simulink model "TosAeb"  (R2021a)
settings: Solver=FixedStepDiscrete  StartTime=0.0  StopTime=inf  FixedStep=0.01  RelTol=1e-3
undefined symbols (not defined in the file or in its folder): ACCModSts, AccSts1, AEB_ObjectMovingOrientation, AEB_ObjectMovingState, CN_BTCActive, CN_CurrentGearR, CN_CurrentGearR_DHT, CN_PTCActive, CN_VDCActive, CN_VSC_EspOff, CN_VSC_FCW_AEB_Error, CN_VSC_FCW_AEB_NoDispay, CN_VSC_FCW_AEB_PerfmDgdtn, CN_VSC_FCW_AEB_TempUnAvlbl, CN_VSC_VehStandstill, CNaccelAvoidMax, CNaccelAvoidMin, CNbrakePedalPress, CNcomfortStrLeftAccelMax, CNcomfortStrLeftAccelMin, CNcomfortStrRightAccelMax, CNcomfortStrRightAccelMin, CNDrvDoorOpen, CNDrvModeEco, CNDrvModeNorm, CNDrvModeOther, CNDrvModeSport, CNDrvSBRreminder, CNNull, CNobjInvalidID, CNPassDoorOpen, CNPassSBRreminder, CNPEBSAccelNegInf, CNPEBSAccelNull, CNPEBSTimeInf, CNPEBSTimeNull, CNSTOPMAX, CNSTOPNULL, CNsubjWidth, CNSwtOFF, CNSwtON, CNTTCMAX, CNTTCNULL, CNVehConfigAEBCycNone, CNVehConfigAEBNone, CNVehConfigAEBPedNone, CNVehConfigFCWNone, CNVehConfigJANone, CollisionSeverity, FALSE, FCWSnvty, JACycObjCalcInfoDefaultParams, JAPedObjCalcInfoDefaultParams, JAVehObjCalcInfoDefaultParams, latCycObjCalcInfoDefaultParams, latPedObjCalcInfoDefaultParams, longObjCalcInfoDefaultParams, ModSts, movingStatus, ObjectType ... (+1112)
libraries: simulink x3031, AEBSFunctionLib x50, Ta_Library x33, AEBSStateMachineLib x23, Mylibrary x12, simulink_extras x7, ObjSelect_lib x3, dspstat3 x2
(part 33 of 102)

== system TosAeb/FcwAebJa_Step_sys/ALG_AEBSystem/ALG_AEBController/AEB_StateMachineCalcApp/VehFCTModStsArb/BrkReadyArb/BrkReadyModStsArb ==
  vehAEBModSts : Inport
  prefillBrkReadyAbor : Inport  Port=2
  ABABrkReadyAbor : Inport  Port=3
  Bus Selector : BusSelector  OutputSignals=vehPrefillModSts
  Bus Selector1 : BusSelector  OutputSignals=vehABAModSts
  Compare To Constant : simulink/Logic and Bit Operations/Compare To Constant  relop=~=  const=ModSts.ActiveState  OutDataTypeStr=boolean
  Compare To Constant1 : simulink/Logic and Bit Operations/Compare To Constant  relop===  const=FALSE  OutDataTypeStr=boolean
  Compare To Constant2 : simulink/Logic and Bit Operations/Compare To Constant  relop=~=  const=ModSts.ActiveState  OutDataTypeStr=boolean
  Compare To Constant3 : simulink/Logic and Bit Operations/Compare To Constant  relop===  const=FALSE  OutDataTypeStr=boolean
  Constant : Constant  Value=ModSts.StandbyState
  Constant1 : Constant  Value=ModSts.StandbyState
  Switch{,1..3} (4 alike) : Switch  Criteria=u2 ~= 0
  prefillModStsArb : Outport
  ABAModStsArb : Outport  Port=2
  wires:
  vehAEBModSts/1 -> Bus Selector1/1, Bus Selector/1
  Bus Selector/1 -> Switch/1, Switch1/1, Compare To Constant/1  "<vehPrefillModSts>"
  Compare To Constant/1 -> Switch/2
  Switch1/1 -> Switch/3
  Compare To Constant1/1 -> Switch1/2
  prefillBrkReadyAbor/1 -> Compare To Constant1/1
  Constant/1 -> Switch1/3
  Switch/1 -> prefillModStsArb/1
  Bus Selector1/1 -> Switch3/1, Switch2/1, Compare To Constant2/1  "<vehABAModSts>"
  Compare To Constant2/1 -> Switch2/2
  ABABrkReadyAbor/1 -> Compare To Constant3/1
  Compare To Constant3/1 -> Switch3/2
  Constant1/1 -> Switch3/3
  Switch3/1 -> Switch2/3
  Switch2/1 -> ABAModStsArb/1

== system TosAeb/FcwAebJa_Step_sys/ALG_AEBSystem/ALG_AEBController/AEB_StateMachineCalcApp/VehFCTModStsArb/BrkReadyArb/BrkReadySuppArb ==
  brkActvCond : Inport
  Compare To Constant : simulink/Logic and Bit Operations/Compare To Constant  relop===  const=TRUE  OutDataTypeStr=boolean
  Constant : Constant  Value=FALSE  OutDataTypeStr=boolean
  prefillBrkReadySuppArb : Outport
  ABABrkReadySuppArb : Outport  Port=2
  wires:
  brkActvCond/1 -> Compare To Constant/1
  Compare To Constant/1 -> prefillBrkReadySuppArb/1
  Constant/1 -> ABABrkReadySuppArb/1

== system TosAeb/FcwAebJa_Step_sys/ALG_AEBSystem/ALG_AEBController/AEB_StateMachineCalcApp/VehFCTModStsArb/WarningArb/BrkReadyAborArb ==
  brkActvCond : Inport
  brkReadyActvCond : Inport  Port=2
  vehAEBModSts : Inport  Port=3
  Bus Selector : BusSelector  OutputSignals=vehPreWarningModSts,vehAcuteWarningModSts
  Bus Selector1 : BusSelector  OutputSignals=vehEBAModSts
  Compare To Constant : simulink/Logic and Bit Operations/Compare To Constant  relop===  const=TRUE  OutDataTypeStr=boolean
  Compare To Constant1 : simulink/Logic and Bit Operations/Compare To Constant  relop===  const=TRUE  OutDataTypeStr=boolean
  Compare To Constant2 : simulink/Logic and Bit Operations/Compare To Constant  relop===  const=ModSts.ActiveState  OutDataTypeStr=boolean
  Compare To Constant3 : simulink/Logic and Bit Operations/Compare To Constant  relop===  const=ModSts.ActiveState  OutDataTypeStr=boolean
  Compare To Constant4 : simulink/Logic and Bit Operations/Compare To Constant  relop===  const=ModSts.ActiveState  OutDataTypeStr=boolean
  Compare To Constant5 : simulink/Logic and Bit Operations/Compare To Constant  relop===  const=TRUE  OutDataTypeStr=boolean
  Logical Operator : Logic  Operator=OR  Inputs=4  AllPortsSameDT=off  OutDataTypeStr=boolean
  latentWarningAborArb : Outport
  preWarningAborArb : Outport  Port=2
  acuteWarningAborArb : Outport  Port=3
  wires:
  brkReadyActvCond/1 -> Compare To Constant1/1
  vehAEBModSts/1 -> Bus Selector/1, Bus Selector1/1
  Bus Selector/1 -> Compare To Constant2/1  "<vehPreWarningModSts>"
  Bus Selector/2 -> Compare To Constant3/1  "<vehAcuteWarningModSts>"
  Compare To Constant1/1 -> Logical Operator/4
  Compare To Constant/1 -> Logical Operator/3
  Compare To Constant3/1 -> Logical Operator/2
  Compare To Constant2/1 -> Logical Operator/1
  Logical Operator/1 -> latentWarningAborArb/1
  Bus Selector1/1 -> Compare To Constant4/1  "<vehEBAModSts>"
  Compare To Constant4/1 -> preWarningAborArb/1
  brkActvCond/1 -> Compare To Constant5/1, Compare To Constant/1
  Compare To Constant5/1 -> acuteWarningAborArb/1

== system TosAeb/FcwAebJa_Step_sys/ALG_AEBSystem/ALG_AEBController/AEB_StateMachineCalcApp/VehFCTModStsArb/WarningArb/WarningActvArbtrtn ==
  latentWarningModStsArb : Inport
  preWarningModStsArb : Inport  Port=2
  acuteWarningModStsArb : Inport  Port=3
  Compare To Constant : simulink/Logic and Bit Operations/Compare To Constant  relop===  const=ModSts.ActiveState  OutDataTypeStr=boolean
  Compare To Constant1 : simulink/Logic and Bit Operations/Compare To Constant  relop===  const=ModSts.ActiveState  OutDataTypeStr=boolean
  Compare To Constant2 : simulink/Logic and Bit Operations/Compare To Constant  relop===  const=ModSts.ActiveState  OutDataTypeStr=boolean
  Logical Operator : Logic  Operator=OR  Inputs=3  AllPortsSameDT=off  OutDataTypeStr=boolean
  WarningActvCond : Outport
  wires:
  Logical Operator/1 -> WarningActvCond/1
  Compare To Constant/1 -> Logical Operator/1
  Compare To Constant1/1 -> Logical Operator/2
  Compare To Constant2/1 -> Logical Operator/3
  latentWarningModStsArb/1 -> Compare To Constant/1
  preWarningModStsArb/1 -> Compare To Constant1/1
  acuteWarningModStsArb/1 -> Compare To Constant2/1

== system TosAeb/FcwAebJa_Step_sys/ALG_AEBSystem/ALG_AEBController/AEB_StateMachineCalcApp/VehFCTModStsArb/WarningArb/WarningModStsArbtrtn ==
  vehAEBModSts : Inport
  latentWarningAborArb : Inport  Port=2
  preWarningAborArb : Inport  Port=3
  acuteWarningAborArb : Inport  Port=4
  Bus Selector : BusSelector  OutputSignals=vehLatentWarningModSts
  Bus Selector1 : BusSelector  OutputSignals=vehPreWarningModSts
  Bus Selector2 : BusSelector  OutputSignals=vehAcuteWarningModSts
  Compare To Constant : simulink/Logic and Bit Operations/Compare To Constant  relop=~=  const=ModSts.ActiveState  OutDataTypeStr=boolean
  Compare To Constant1 : simulink/Logic and Bit Operations/Compare To Constant  relop===  const=FALSE  OutDataTypeStr=boolean
  Compare To Constant2 : simulink/Logic and Bit Operations/Compare To Constant  relop=~=  const=ModSts.ActiveState  OutDataTypeStr=boolean
  Compare To Constant3 : simulink/Logic and Bit Operations/Compare To Constant  relop===  const=FALSE  OutDataTypeStr=boolean
  Compare To Constant4 : simulink/Logic and Bit Operations/Compare To Constant  relop=~=  const=ModSts.ActiveState  OutDataTypeStr=boolean
  Compare To Constant5 : simulink/Logic and Bit Operations/Compare To Constant  relop===  const=FALSE  OutDataTypeStr=boolean
  Constant : Constant  Value=ModSts.StandbyState
  Constant1 : Constant  Value=ModSts.StandbyState
  Constant2 : Constant  Value=ModSts.StandbyState
  Switch{,1..5} (6 alike) : Switch  Criteria=u2 ~= 0
  latentWarningModStsArb : Outport
  preWarningModStsArb : Outport  Port=2
  acuteWarningModStsArb : Outport  Port=3
  wires:
  Switch2/1 -> preWarningModStsArb/1
  Switch3/1 -> Switch2/3
  Constant1/1 -> Switch3/3
  Compare To Constant3/1 -> Switch3/2
  preWarningAborArb/1 -> Compare To Constant3/1
  Compare To Constant2/1 -> Switch2/2
  Bus Selector1/1 -> Compare To Constant2/1, Switch2/1, Switch3/1  "<vehPreWarningModSts>"
  Switch/1 -> latentWarningModStsArb/1
  Constant/1 -> Switch1/3
  latentWarningAborArb/1 -> Compare To Constant1/1
  Compare To Constant1/1 -> Switch1/2
  Switch1/1 -> Switch/3
  Compare To Constant/1 -> Switch/2
  Bus Selector/1 -> Compare To Constant/1, Switch1/1, Switch/1  "<vehLatentWarningModSts>"
  vehAEBModSts/1 -> Bus Selector/1, Bus Selector2/1, Bus Selector1/1
  Bus Selector2/1 -> Switch5/1, Switch4/1, Compare To Constant4/1  "<vehAcuteWarningModSts>"
  Compare To Constant4/1 -> Switch4/2
  acuteWarningAborArb/1 -> Compare To Constant5/1
  Compare To Constant5/1 -> Switch5/2
  Constant2/1 -> Switch5/3
  Switch5/1 -> Switch4/3
  Switch4/1 -> acuteWarningModStsArb/1

== system TosAeb/FcwAebJa_Step_sys/ALG_AEBSystem/ALG_AEBController/AEB_StateMachineCalcApp/VehFCTModStsArb/WarningArb/WarningSuppArb ==
  vehAEBModSts : Inport
  brkReadyActvCond : Inport  Port=2
  brkActvCond : Inport  Port=3
  Bus Selector : BusSelector  OutputSignals=vehPreWarningModSts,vehLatentWarningModSts
  Compare To Constant{,1,4..5} (4 alike) : simulink/Logic and Bit Operations/Compare To Constant  relop===  const=TRUE  OutDataTypeStr=boolean
  Compare To Constant2 : simulink/Logic and Bit Operations/Compare To Constant  relop===  const=ModSts.ActiveState  OutDataTypeStr=boolean
  Compare To Constant3 : simulink/Logic and Bit Operations/Compare To Constant  relop===  const=ModSts.ActiveState  OutDataTypeStr=boolean
  Logical Operator : Logic  Operator=OR  Inputs=4  AllPortsSameDT=off  OutDataTypeStr=boolean
  latentWarningSuppArb : Outport
  prewarningSuppArb : Outport  Port=2
  acutewarningSuppArb : Outport  Port=3
  wires:
  brkReadyActvCond/1 -> Compare To Constant/1
  brkActvCond/1 -> Compare To Constant5/1, Compare To Constant4/1, Compare To Constant1/1
  vehAEBModSts/1 -> Bus Selector/1
  Bus Selector/1 -> Compare To Constant2/1  "<vehPreWarningModSts>"
  Bus Selector/2 -> Compare To Constant3/1  "<vehLatentWarningModSts>"
  Compare To Constant2/1 -> Logical Operator/1
  Compare To Constant3/1 -> Logical Operator/2
  Compare To Constant/1 -> Logical Operator/3
  Compare To Constant1/1 -> Logical Operator/4
  Logical Operator/1 -> latentWarningSuppArb/1
  Compare To Constant4/1 -> prewarningSuppArb/1
  Compare To Constant5/1 -> acutewarningSuppArb/1

== system TosAeb/FcwAebJa_Step_sys/ALG_AEBSystem/ALG_AEBController/AEB_VehicleStateCalcApp/CycSuppAborCondtionCalc/CycAEBfullSuppAborConditonCalc/VehStateCalcCycAEBfullAborCalc ==
  VSCAEB_PedResp : Inport
  FCW_AEB_PedFctSts : Inport  Port=2
  vehAssociatedSysStateSuppCond : Inport  Port=3
  vehUnstableStateFlg : Inport  Port=4
  Compare To Constant : simulink/Logic and Bit Operations/Compare To Constant  relop===  const=CNSwtOFF  OutDataTypeStr=boolean
  Compare To Constant2 : simulink/Logic and Bit Operations/Compare To Constant  relop===  const=TRUE  OutDataTypeStr=boolean
  Compare To Constant3 : simulink/Logic and Bit Operations/Compare To Constant  relop===  const=TRUE  OutDataTypeStr=boolean
  Compare To Constant4 : simulink/Logic and Bit Operations/Compare To Constant  relop===  const=CN_VSC_FCW_AEB_Error  OutDataTypeStr=boolean
  Compare To Constant8 : simulink/Logic and Bit Operations/Compare To Constant  relop===  const=CN_VSC_FCW_AEB_PerfmDgdtn  OutDataTypeStr=boolean
  Compare To Constant9 : simulink/Logic and Bit Operations/Compare To Constant  relop===  const=CN_VSC_FCW_AEB_TempUnAvlbl  OutDataTypeStr=boolean
  Logical Operator : Logic  Operator=OR  Inputs=3  AllPortsSameDT=off  OutDataTypeStr=boolean
  Logical Operator1 : Logic  Operator=OR  Inputs=4  AllPortsSameDT=off  OutDataTypeStr=boolean
  VSCCycAEBfullAborCond : Outport
  wires:
  FCW_AEB_PedFctSts/1 -> Compare To Constant4/1, Compare To Constant8/1, Compare To Constant9/1
  Logical Operator/1 -> Logical Operator1/2
  Compare To Constant9/1 -> Logical Operator/3
  Compare To Constant8/1 -> Logical Operator/2
  Compare To Constant4/1 -> Logical Operator/1
  vehUnstableStateFlg/1 -> Compare To Constant3/1
  Logical Operator1/1 -> VSCCycAEBfullAborCond/1
  vehAssociatedSysStateSuppCond/1 -> Compare To Constant2/1
  Compare To Constant/1 -> Logical Operator1/1
  VSCAEB_PedResp/1 -> Compare To Constant/1
  Compare To Constant2/1 -> Logical Operator1/3
  Compare To Constant3/1 -> Logical Operator1/4

== system TosAeb/FcwAebJa_Step_sys/ALG_AEBSystem/ALG_AEBController/AEB_VehicleStateCalcApp/CycSuppAborCondtionCalc/CycAEBfullSuppAborConditonCalc/VehStateCalcCycAEBfullSuppCalc ==
  VSCAEB_PedResp : Inport
  FCW_AEB_PedFctSts : Inport  Port=2
  vehUnstableStateFlg : Inport  Port=3
  vehAssociatedSysStateSuppCond : Inport  Port=4
  "vehConfigAEBCyc " : Inport  Port=5
  blockTimeBrakeReady : Inport  Port=6
  Compare To Constant : simulink/Logic and Bit Operations/Compare To Constant  relop===  const=CNSwtOFF  OutDataTypeStr=boolean
  Compare To Constant1 : simulink/Logic and Bit Operations/Compare To Constant  relop===  const=CN_VSC_FCW_AEB_Error  OutDataTypeStr=boolean
  Compare To Constant3 : simulink/Logic and Bit Operations/Compare To Constant  relop===  const=CNVehConfigAEBCycNone  OutDataTypeStr=boolean
  Compare To Constant4 : simulink/Logic and Bit Operations/Compare To Constant  relop=>  const=CNNull  OutDataTypeStr=boolean
  Compare To Constant5 : simulink/Logic and Bit Operations/Compare To Constant  relop===  const=TRUE  OutDataTypeStr=boolean
  Compare To Constant6 : simulink/Logic and Bit Operations/Compare To Constant  relop===  const=TRUE  OutDataTypeStr=boolean
  Compare To Constant7 : simulink/Logic and Bit Operations/Compare To Constant  relop===  const=CNSwtOFF  OutDataTypeStr=boolean
  Compare To Constant8 : simulink/Logic and Bit Operations/Compare To Constant  relop===  const=CN_VSC_FCW_AEB_PerfmDgdtn  OutDataTypeStr=boolean
  Compare To Constant9 : simulink/Logic and Bit Operations/Compare To Constant  relop===  const=CN_VSC_FCW_AEB_TempUnAvlbl  OutDataTypeStr=boolean
  Constant : Constant  Value=P_AEB_InterSwtSts4CycAEBfull
  Logical Operator : Logic  Operator=OR  Inputs=3  AllPortsSameDT=off  OutDataTypeStr=boolean
  Logical Operator1 : Logic  Operator=OR  Inputs=7  AllPortsSameDT=off  OutDataTypeStr=boolean
  VSCCycAEBfullSuppCond : Outport
  wires:
  FCW_AEB_PedFctSts/1 -> Compare To Constant1/1, Compare To Constant8/1, Compare To Constant9/1
  Compare To Constant1/1 -> Logical Operator/1
  Compare To Constant9/1 -> Logical Operator/3
  Compare To Constant8/1 -> Logical Operator/2
  Compare To Constant7/1 -> Logical Operator1/7
  Constant/1 -> Compare To Constant7/1
  VSCAEB_PedResp/1 -> Compare To Constant/1
  Compare To Constant/1 -> Logical Operator1/1
  Compare To Constant3/1 -> Logical Operator1/5
  vehAssociatedSysStateSuppCond/1 -> Compare To Constant5/1
  blockTimeBrakeReady/1 -> Compare To Constant4/1
  Logical Operator/1 -> Logical Operator1/2
  vehUnstableStateFlg/1 -> Compare To Constant6/1
  Logical Operator1/1 -> VSCCycAEBfullSuppCond/1
  "vehConfigAEBCyc "/1 -> Compare To Constant3/1
  Compare To Constant4/1 -> Logical Operator1/6
  Compare To Constant5/1 -> Logical Operator1/4
  Compare To Constant6/1 -> Logical Operator1/3

== system TosAeb/FcwAebJa_Step_sys/ALG_AEBSystem/ALG_AEBController/AEB_VehicleStateCalcApp/CycSuppAborCondtionCalc/CycAEBpartSuppAborConditonCalc/VehStateCalcCycAEBpartAborCalc ==
  AEB_PedResp : Inport
  FCW_AEB_PedFctSts : Inport  Port=2
  vehAssociatedSysStateSuppCond : Inport  Port=3
  vehUnstableStateFlg : Inport  Port=4
  Compare To Constant : simulink/Logic and Bit Operations/Compare To Constant  relop===  const=CNSwtOFF  OutDataTypeStr=boolean
  Compare To Constant2 : simulink/Logic and Bit Operations/Compare To Constant  relop===  const=TRUE  OutDataTypeStr=boolean
  Compare To Constant3 : simulink/Logic and Bit Operations/Compare To Constant  relop===  const=TRUE  OutDataTypeStr=boolean
  Compare To Constant6 : simulink/Logic and Bit Operations/Compare To Constant  relop===  const=CN_VSC_FCW_AEB_Error  OutDataTypeStr=boolean
  Compare To Constant8 : simulink/Logic and Bit Operations/Compare To Constant  relop===  const=CN_VSC_FCW_AEB_PerfmDgdtn  OutDataTypeStr=boolean
  Compare To Constant9 : simulink/Logic and Bit Operations/Compare To Constant  relop===  const=CN_VSC_FCW_AEB_TempUnAvlbl  OutDataTypeStr=boolean
  Logical Operator : Logic  Operator=OR  Inputs=3  AllPortsSameDT=off  OutDataTypeStr=boolean
  Logical Operator1 : Logic  Operator=OR  Inputs=4  AllPortsSameDT=off  OutDataTypeStr=boolean
  VSCAEBpartAborCond : Outport
  wires:
  FCW_AEB_PedFctSts/1 -> Compare To Constant6/1, Compare To Constant8/1, Compare To Constant9/1
  Compare To Constant6/1 -> Logical Operator/1
  Compare To Constant9/1 -> Logical Operator/3
  Compare To Constant8/1 -> Logical Operator/2
  AEB_PedResp/1 -> Compare To Constant/1
  Compare To Constant/1 -> Logical Operator1/1
  vehAssociatedSysStateSuppCond/1 -> Compare To Constant2/1
  Logical Operator/1 -> Logical Operator1/2
  Logical Operator1/1 -> VSCAEBpartAborCond/1
  vehUnstableStateFlg/1 -> Compare To Constant3/1
  Compare To Constant2/1 -> Logical Operator1/3
  Compare To Constant3/1 -> Logical Operator1/4

== system TosAeb/FcwAebJa_Step_sys/ALG_AEBSystem/ALG_AEBController/AEB_VehicleStateCalcApp/CycSuppAborCondtionCalc/CycAEBpartSuppAborConditonCalc/VehStateCalcCycAEBpartSuppCalc ==
  AEB_PedResp : Inport
  FCW_AEB_PedFctSts : Inport  Port=2
  vehUnstableStateFlg : Inport  Port=3
  vehAssociatedSysStateSuppCond : Inport  Port=4
  "vehConfigAEBCyc " : Inport  Port=5
  blockTimeBrake : Inport  Port=6
  Compare To Constant : simulink/Logic and Bit Operations/Compare To Constant  relop===  const=CNSwtOFF  OutDataTypeStr=boolean
  Compare To Constant2 : simulink/Logic and Bit Operations/Compare To Constant  relop===  const=TRUE  OutDataTypeStr=boolean
  Compare To Constant3 : simulink/Logic and Bit Operations/Compare To Constant  relop===  const=CNVehConfigAEBCycNone  OutDataTypeStr=boolean
  Compare To Constant4 : simulink/Logic and Bit Operations/Compare To Constant  relop=>  const=CNNull  OutDataTypeStr=boolean
  Compare To Constant5 : simulink/Logic and Bit Operations/Compare To Constant  relop===  const=TRUE  OutDataTypeStr=boolean
  Compare To Constant6 : simulink/Logic and Bit Operations/Compare To Constant  relop===  const=CN_VSC_FCW_AEB_Error  OutDataTypeStr=boolean
  Compare To Constant7 : simulink/Logic and Bit Operations/Compare To Constant  relop===  const=CNSwtOFF  OutDataTypeStr=boolean
  Compare To Constant8 : simulink/Logic and Bit Operations/Compare To Constant  relop===  const=CN_VSC_FCW_AEB_PerfmDgdtn  OutDataTypeStr=boolean
  Compare To Constant9 : simulink/Logic and Bit Operations/Compare To Constant  relop===  const=CN_VSC_FCW_AEB_TempUnAvlbl  OutDataTypeStr=boolean
  Constant : Constant  Value=P_AEB_InterSwtSts4CycAEBpart
  Logical Operator : Logic  Operator=OR  Inputs=3  AllPortsSameDT=off  OutDataTypeStr=boolean
  Logical Operator1 : Logic  Operator=OR  Inputs=7  AllPortsSameDT=off  OutDataTypeStr=boolean
  VSCAEBpartSuppCond : Outport
  wires:
  FCW_AEB_PedFctSts/1 -> Compare To Constant6/1, Compare To Constant8/1, Compare To Constant9/1
  Logical Operator/1 -> Logical Operator1/2
  Compare To Constant6/1 -> Logical Operator/1
  Compare To Constant8/1 -> Logical Operator/2
  Compare To Constant9/1 -> Logical Operator/3
  Compare To Constant7/1 -> Logical Operator1/7
  Constant/1 -> Compare To Constant7/1
  Compare To Constant4/1 -> Logical Operator1/6
  "vehConfigAEBCyc "/1 -> Compare To Constant3/1
  Logical Operator1/1 -> VSCAEBpartSuppCond/1
  vehUnstableStateFlg/1 -> Compare To Constant5/1
  blockTimeBrake/1 -> Compare To Constant4/1
  vehAssociatedSysStateSuppCond/1 -> Compare To Constant2/1
  Compare To Constant3/1 -> Logical Operator1/5
  Compare To Constant/1 -> Logical Operator1/1
  AEB_PedResp/1 -> Compare To Constant/1
  Compare To Constant2/1 -> Logical Operator1/4
  Compare To Constant5/1 -> Logical Operator1/3

== system TosAeb/FcwAebJa_Step_sys/ALG_AEBSystem/ALG_AEBController/AEB_VehicleStateCalcApp/CycSuppAborCondtionCalc/CycPrefillSuppAborConditonCalc/VehStateCalcPrefillAborCalc ==
  AEB_PedResp : Inport
  FCW_AEB_PedFctSts : Inport  Port=2
  vehUnstableStateFlg : Inport  Port=3
  vehAssociatedSysStateSuppCond : Inport  Port=4
  Compare To Constant : simulink/Logic and Bit Operations/Compare To Constant  relop===  const=CNSwtOFF  OutDataTypeStr=boolean
  Compare To Constant2 : simulink/Logic and Bit Operations/Compare To Constant  relop===  const=TRUE  OutDataTypeStr=boolean
  Compare To Constant3 : simulink/Logic and Bit Operations/Compare To Constant  relop===  const=TRUE  OutDataTypeStr=boolean
  Compare To Constant4 : simulink/Logic and Bit Operations/Compare To Constant  relop===  const=CN_VSC_FCW_AEB_Error  OutDataTypeStr=boolean
  Compare To Constant5 : simulink/Logic and Bit Operations/Compare To Constant  relop===  const=CN_VSC_FCW_AEB_PerfmDgdtn  OutDataTypeStr=boolean
  Compare To Constant8 : simulink/Logic and Bit Operations/Compare To Constant  relop===  const=CN_VSC_FCW_AEB_TempUnAvlbl  OutDataTypeStr=boolean
  Logical Operator : Logic  Operator=OR  Inputs=3  AllPortsSameDT=off  OutDataTypeStr=boolean
  Logical Operator1 : Logic  Operator=OR  Inputs=4  AllPortsSameDT=off  OutDataTypeStr=boolean
  VSCCycPrefillAborCond : Outport
  wires:
  FCW_AEB_PedFctSts/1 -> Compare To Constant4/1, Compare To Constant5/1, Compare To Constant8/1
  Logical Operator/1 -> Logical Operator1/2
  Compare To Constant4/1 -> Logical Operator/1
  Compare To Constant5/1 -> Logical Operator/2
  Compare To Constant8/1 -> Logical Operator/3
  Logical Operator1/1 -> VSCCycPrefillAborCond/1
  vehUnstableStateFlg/1 -> Compare To Constant2/1
  vehAssociatedSysStateSuppCond/1 -> Compare To Constant3/1
  Compare To Constant/1 -> Logical Operator1/1
  AEB_PedResp/1 -> Compare To Constant/1
  Compare To Constant2/1 -> Logical Operator1/3
  Compare To Constant3/1 -> Logical Operator1/4

== system TosAeb/FcwAebJa_Step_sys/ALG_AEBSystem/ALG_AEBController/AEB_VehicleStateCalcApp/CycSuppAborCondtionCalc/CycPrefillSuppAborConditonCalc/VehStateCalcPrefillSuppCalc ==
  VSCAEB_PedResp : Inport
  FCW_AEB_PedFctSts : Inport  Port=2
  vehUnstableStateFlg : Inport  Port=3
  vehAssociatedSysStateSuppCond : Inport  Port=4
  "vehConfigAEBCyc " : Inport  Port=5
  blockTimeBrakeReady : Inport  Port=6
  Compare To Constant : simulink/Logic and Bit Operations/Compare To Constant  relop===  const=CNSwtOFF  OutDataTypeStr=boolean
  Compare To Constant1 : simulink/Logic and Bit Operations/Compare To Constant  relop===  const=CN_VSC_FCW_AEB_Error  OutDataTypeStr=boolean
  Compare To Constant2 : simulink/Logic and Bit Operations/Compare To Constant  relop===  const=TRUE  OutDataTypeStr=boolean
  Compare To Constant3 : simulink/Logic and Bit Operations/Compare To Constant  relop===  const=CNVehConfigAEBCycNone  OutDataTypeStr=boolean
  Compare To Constant4 : simulink/Logic and Bit Operations/Compare To Constant  relop=>  const=CNNull  OutDataTypeStr=boolean
  Compare To Constant5 : simulink/Logic and Bit Operations/Compare To Constant  relop===  const=TRUE  OutDataTypeStr=boolean
  Compare To Constant6 : simulink/Logic and Bit Operations/Compare To Constant  relop===  const=CN_VSC_FCW_AEB_PerfmDgdtn  OutDataTypeStr=boolean
  Compare To Constant7 : simulink/Logic and Bit Operations/Compare To Constant  relop===  const=CNSwtOFF  OutDataTypeStr=boolean
  Compare To Constant8 : simulink/Logic and Bit Operations/Compare To Constant  relop===  const=CN_VSC_FCW_AEB_TempUnAvlbl  OutDataTypeStr=boolean
  Constant : Constant  Value=P_AEB_InterSwtSts4CycPrefill
  Logical Operator : Logic  Operator=OR  Inputs=3  AllPortsSameDT=off  OutDataTypeStr=boolean
  Logical Operator1 : Logic  Operator=OR  Inputs=6  AllPortsSameDT=off  OutDataTypeStr=boolean
  Terminator : Terminator
  VSCCycPrefillSuppCond : Outport
  wires:
  FCW_AEB_PedFctSts/1 -> Compare To Constant1/1, Compare To Constant6/1, Compare To Constant8/1
  Compare To Constant1/1 -> Logical Operator/1
  Compare To Constant6/1 -> Logical Operator/2
  Compare To Constant8/1 -> Logical Operator/3
  Compare To Constant7/1 -> Logical Operator1/6
  Constant/1 -> Compare To Constant7/1
  VSCAEB_PedResp/1 -> Compare To Constant/1
  Compare To Constant/1 -> Logical Operator1/1
  Compare To Constant3/1 -> Logical Operator1/5
  vehAssociatedSysStateSuppCond/1 -> Compare To Constant2/1
  blockTimeBrakeReady/1 -> Compare To Constant4/1
  Logical Operator/1 -> Logical Operator1/2
  vehUnstableStateFlg/1 -> Compare To Constant5/1
  Logical Operator1/1 -> VSCCycPrefillSuppCond/1
  "vehConfigAEBCyc "/1 -> Compare To Constant3/1
  Compare To Constant4/1 -> Terminator/1
  Compare To Constant2/1 -> Logical Operator1/4
  Compare To Constant5/1 -> Logical Operator1/3

== system TosAeb/FcwAebJa_Step_sys/ALG_AEBSystem/ALG_AEBController/AEB_VehicleStateCalcApp/JaSuppAborCondtionCalc/JACycAEBSuppAborConditonCalc/VehStateCalcJAAEBAborCalc ==
  AEB_PedResp : Inport
  FCW_AEB_JAFctSts : Inport  Port=2
  vehAssociatedSysStateSuppCond : Inport  Port=3
  vehUnstableStateFlg : Inport  Port=4
  Compare To Constant : simulink/Logic and Bit Operations/Compare To Constant  relop===  const=CNSwtOFF  OutDataTypeStr=boolean
  Compare To Constant1 : simulink/Logic and Bit Operations/Compare To Constant  relop===  const=CN_VSC_FCW_AEB_Error  OutDataTypeStr=boolean
  Compare To Constant2 : simulink/Logic and Bit Operations/Compare To Constant  relop===  const=TRUE  OutDataTypeStr=boolean
  Compare To Constant3 : simulink/Logic and Bit Operations/Compare To Constant  relop===  const=TRUE  OutDataTypeStr=boolean
  Compare To Constant8 : simulink/Logic and Bit Operations/Compare To Constant  relop===  const=CN_VSC_FCW_AEB_PerfmDgdtn  OutDataTypeStr=boolean
  Compare To Constant9 : simulink/Logic and Bit Operations/Compare To Constant  relop===  const=CN_VSC_FCW_AEB_TempUnAvlbl  OutDataTypeStr=boolean
  Logical Operator : Logic  Operator=OR  Inputs=3  AllPortsSameDT=off  OutDataTypeStr=boolean
  Logical Operator1 : Logic  Operator=OR  Inputs=4  AllPortsSameDT=off  OutDataTypeStr=boolean
  VSC_JACycAEBfullAborCond : Outport
  wires:
  FCW_AEB_JAFctSts/1 -> Compare To Constant1/1, Compare To Constant8/1, Compare To Constant9/1
  Logical Operator/1 -> Logical Operator1/2
  Compare To Constant8/1 -> Logical Operator/2
  Compare To Constant1/1 -> Logical Operator/1
  Compare To Constant9/1 -> Logical Operator/3
  Compare To Constant3/1 -> Logical Operator1/4
  Compare To Constant2/1 -> Logical Operator1/3
  vehUnstableStateFlg/1 -> Compare To Constant3/1
  Logical Operator1/1 -> VSC_JACycAEBfullAborCond/1
  vehAssociatedSysStateSuppCond/1 -> Compare To Constant2/1
  Compare To Constant/1 -> Logical Operator1/1
  AEB_PedResp/1 -> Compare To Constant/1

== system TosAeb/FcwAebJa_Step_sys/ALG_AEBSystem/ALG_AEBController/AEB_VehicleStateCalcApp/JaSuppAborCondtionCalc/JACycAEBSuppAborConditonCalc/VehStateCalcJAAEBSuppCalc ==
  AEB_PedResp : Inport
  FCW_AEB_JAFctSts : Inport  Port=2
  vehUnstableStateFlg : Inport  Port=3
  vehAssociatedSysStateSuppCond : Inport  Port=4
  "VehicleConfigAEBPed " : Inport  Port=5
  blockTimeBrake : Inport  Port=6
  Compare To Constant : simulink/Logic and Bit Operations/Compare To Constant  relop===  const=CNSwtOFF  OutDataTypeStr=boolean
  Compare To Constant1 : simulink/Logic and Bit Operations/Compare To Constant  relop===  const=CN_VSC_FCW_AEB_Error  OutDataTypeStr=boolean
  Compare To Constant2 : simulink/Logic and Bit Operations/Compare To Constant  relop===  const=TRUE  OutDataTypeStr=boolean
  Compare To Constant3 : simulink/Logic and Bit Operations/Compare To Constant  relop===  const=CNVehConfigAEBPedNone  OutDataTypeStr=boolean
  Compare To Constant4 : simulink/Logic and Bit Operations/Compare To Constant  relop=>  const=CNNull  OutDataTypeStr=boolean
  Compare To Constant5 : simulink/Logic and Bit Operations/Compare To Constant  relop===  const=TRUE  OutDataTypeStr=boolean
  Compare To Constant7 : simulink/Logic and Bit Operations/Compare To Constant  relop===  const=CNSwtOFF  OutDataTypeStr=boolean
  Compare To Constant8 : simulink/Logic and Bit Operations/Compare To Constant  relop===  const=CN_VSC_FCW_AEB_PerfmDgdtn  OutDataTypeStr=boolean
  Compare To Constant9 : simulink/Logic and Bit Operations/Compare To Constant  relop===  const=CN_VSC_FCW_AEB_TempUnAvlbl  OutDataTypeStr=boolean
  Constant : Constant  Value=P_AEB_InterSwtSts4JACycAEBfull
  Logical Operator : Logic  Operator=OR  Inputs=3  AllPortsSameDT=off  OutDataTypeStr=boolean
  Logical Operator1 : Logic  Operator=OR  Inputs=7  AllPortsSameDT=off  OutDataTypeStr=boolean
  VSC_JACycAEBfullSuppCond : Outport
  wires:
  FCW_AEB_JAFctSts/1 -> Compare To Constant1/1, Compare To Constant8/1, Compare To Constant9/1
  Logical Operator/1 -> Logical Operator1/2
  Compare To Constant8/1 -> Logical Operator/2
  Compare To Constant1/1 -> Logical Operator/1
  Compare To Constant9/1 -> Logical Operator/3
  Compare To Constant7/1 -> Logical Operator1/7
  Constant/1 -> Compare To Constant7/1
  Compare To Constant5/1 -> Logical Operator1/4
  Compare To Constant2/1 -> Logical Operator1/3
  AEB_PedResp/1 -> Compare To Constant/1
  Compare To Constant/1 -> Logical Operator1/1
  Compare To Constant3/1 -> Logical Operator1/5
  vehAssociatedSysStateSuppCond/1 -> Compare To Constant5/1
  blockTimeBrake/1 -> Compare To Constant4/1
  vehUnstableStateFlg/1 -> Compare To Constant2/1
  Logical Operator1/1 -> VSC_JACycAEBfullSuppCond/1
  "VehicleConfigAEBPed "/1 -> Compare To Constant3/1
  Compare To Constant4/1 -> Logical Operator1/6

== system TosAeb/FcwAebJa_Step_sys/ALG_AEBSystem/ALG_AEBController/AEB_VehicleStateCalcApp/JaSuppAborCondtionCalc/JACycPrefillSuppAborConditonCalc/VehStateCalcJAPrefillAborCalc ==
  AEB_PedResp : Inport
  FCW_AEB_JAFctSts : Inport  Port=2
  vehAssociatedSysStateSuppCond : Inport  Port=3
  vehUnstableStateFlg : Inport  Port=4
  Compare To Constant : simulink/Logic and Bit Operations/Compare To Constant  relop===  const=CNSwtOFF  OutDataTypeStr=boolean
  Compare To Constant1 : simulink/Logic and Bit Operations/Compare To Constant  relop===  const=CN_VSC_FCW_AEB_Error  OutDataTypeStr=boolean
  Compare To Constant2 : simulink/Logic and Bit Operations/Compare To Constant  relop===  const=TRUE  OutDataTypeStr=boolean
  Compare To Constant3 : simulink/Logic and Bit Operations/Compare To Constant  relop===  const=TRUE  OutDataTypeStr=boolean
  Compare To Constant8 : simulink/Logic and Bit Operations/Compare To Constant  relop===  const=CN_VSC_FCW_AEB_PerfmDgdtn  OutDataTypeStr=boolean
  Compare To Constant9 : simulink/Logic and Bit Operations/Compare To Constant  relop===  const=CN_VSC_FCW_AEB_TempUnAvlbl  OutDataTypeStr=boolean
  Logical Operator : Logic  Operator=OR  Inputs=3  AllPortsSameDT=off  OutDataTypeStr=boolean
  Logical Operator1 : Logic  Operator=OR  Inputs=4  AllPortsSameDT=off  OutDataTypeStr=boolean
  VSC_JACycPrefillAborCond : Outport
  wires:
  FCW_AEB_JAFctSts/1 -> Compare To Constant1/1, Compare To Constant8/1, Compare To Constant9/1
  Logical Operator/1 -> Logical Operator1/2
  Compare To Constant8/1 -> Logical Operator/2
  Compare To Constant1/1 -> Logical Operator/1
  Compare To Constant9/1 -> Logical Operator/3
  Compare To Constant3/1 -> Logical Operator1/4
  Compare To Constant2/1 -> Logical Operator1/3
  AEB_PedResp/1 -> Compare To Constant/1
  Compare To Constant/1 -> Logical Operator1/1
  vehAssociatedSysStateSuppCond/1 -> Compare To Constant2/1
  Logical Operator1/1 -> VSC_JACycPrefillAborCond/1
  vehUnstableStateFlg/1 -> Compare To Constant3/1

== system TosAeb/FcwAebJa_Step_sys/ALG_AEBSystem/ALG_AEBController/AEB_VehicleStateCalcApp/JaSuppAborCondtionCalc/JACycPrefillSuppAborConditonCalc/VehStateCalcJAPrefillSuppCalc ==
  AEB_PedResp : Inport
  FCW_AEB_JAFctSts : Inport  Port=2
  vehUnstableStateFlg : Inport  Port=3
  vehAssociatedSysStateSuppCond : Inport  Port=4
  "VehicleConfigAEBPed " : Inport  Port=5
  blockTimeBrakeReady : Inport  Port=6
  Compare To Constant : simulink/Logic and Bit Operations/Compare To Constant  relop===  const=CNSwtOFF  OutDataTypeStr=boolean
  Compare To Constant1 : simulink/Logic and Bit Operations/Compare To Constant  relop===  const=CN_VSC_FCW_AEB_Error  OutDataTypeStr=boolean
  Compare To Constant3 : simulink/Logic and Bit Operations/Compare To Constant  relop===  const=CNVehConfigAEBPedNone  OutDataTypeStr=boolean
  Compare To Constant4 : simulink/Logic and Bit Operations/Compare To Constant  relop=>  const=CNNull  OutDataTypeStr=boolean
  Compare To Constant5 : simulink/Logic and Bit Operations/Compare To Constant  relop===  const=TRUE  OutDataTypeStr=boolean
  Compare To Constant6 : simulink/Logic and Bit Operations/Compare To Constant  relop===  const=TRUE  OutDataTypeStr=boolean
  Compare To Constant7 : simulink/Logic and Bit Operations/Compare To Constant  relop===  const=CNSwtOFF  OutDataTypeStr=boolean
  Compare To Constant8 : simulink/Logic and Bit Operations/Compare To Constant  relop===  const=CN_VSC_FCW_AEB_PerfmDgdtn  OutDataTypeStr=boolean
  Compare To Constant9 : simulink/Logic and Bit Operations/Compare To Constant  relop===  const=CN_VSC_FCW_AEB_TempUnAvlbl  OutDataTypeStr=boolean
  Constant : Constant  Value=P_AEB_InterSwtSts4JACycPrefill
  Logical Operator : Logic  Operator=OR  Inputs=3  AllPortsSameDT=off  OutDataTypeStr=boolean
  Logical Operator1 : Logic  Operator=OR  Inputs=6  AllPortsSameDT=off  OutDataTypeStr=boolean
  Terminator : Terminator
  VSC_JACycPrefillSuppCond : Outport
  wires:
  FCW_AEB_JAFctSts/1 -> Compare To Constant1/1, Compare To Constant8/1, Compare To Constant9/1
  Logical Operator/1 -> Logical Operator1/2
  Compare To Constant8/1 -> Logical Operator/2
  Compare To Constant1/1 -> Logical Operator/1
  Compare To Constant9/1 -> Logical Operator/3
  Compare To Constant7/1 -> Logical Operator1/6
  Constant/1 -> Compare To Constant7/1
  Compare To Constant6/1 -> Logical Operator1/3
  Compare To Constant5/1 -> Logical Operator1/4
  Compare To Constant4/1 -> Terminator/1
  "VehicleConfigAEBPed "/1 -> Compare To Constant3/1
  Logical Operator1/1 -> VSC_JACycPrefillSuppCond/1
  vehUnstableStateFlg/1 -> Compare To Constant6/1
  blockTimeBrakeReady/1 -> Compare To Constant4/1
  vehAssociatedSysStateSuppCond/1 -> Compare To Constant5/1
  Compare To Constant3/1 -> Logical Operator1/5
  Compare To Constant/1 -> Logical Operator1/1
  AEB_PedResp/1 -> Compare To Constant/1

== system TosAeb/FcwAebJa_Step_sys/ALG_AEBSystem/ALG_AEBController/AEB_VehicleStateCalcApp/JaSuppAborCondtionCalc/JAPedAEBSuppAborConditonCalc/VehStateCalcJAAEBAborCalc ==
  AEB_PedResp : Inport
  FCW_AEB_JAFctSts : Inport  Port=2
  vehAssociatedSysStateSuppCond : Inport  Port=3
  vehUnstableStateFlg : Inport  Port=4
  Compare To Constant : simulink/Logic and Bit Operations/Compare To Constant  relop===  const=CNSwtOFF  OutDataTypeStr=boolean
  Compare To Constant1 : simulink/Logic and Bit Operations/Compare To Constant  relop===  const=CN_VSC_FCW_AEB_Error  OutDataTypeStr=boolean
  Compare To Constant2 : simulink/Logic and Bit Operations/Compare To Constant  relop===  const=TRUE  OutDataTypeStr=boolean
  Compare To Constant3 : simulink/Logic and Bit Operations/Compare To Constant  relop===  const=TRUE  OutDataTypeStr=boolean
  Compare To Constant8 : simulink/Logic and Bit Operations/Compare To Constant  relop===  const=CN_VSC_FCW_AEB_PerfmDgdtn  OutDataTypeStr=boolean
  Compare To Constant9 : simulink/Logic and Bit Operations/Compare To Constant  relop===  const=CN_VSC_FCW_AEB_TempUnAvlbl  OutDataTypeStr=boolean
  Logical Operator : Logic  Operator=OR  Inputs=3  AllPortsSameDT=off  OutDataTypeStr=boolean
  Logical Operator1 : Logic  Operator=OR  Inputs=4  AllPortsSameDT=off  OutDataTypeStr=boolean
  VSCJAPedAEBfullAborCond : Outport
  wires:
  AEB_PedResp/1 -> Compare To Constant/1
  Compare To Constant/1 -> Logical Operator1/1
  vehAssociatedSysStateSuppCond/1 -> Compare To Constant2/1
  Logical Operator1/1 -> VSCJAPedAEBfullAborCond/1
  vehUnstableStateFlg/1 -> Compare To Constant3/1
  Compare To Constant2/1 -> Logical Operator1/3
  Compare To Constant3/1 -> Logical Operator1/4
  Compare To Constant9/1 -> Logical Operator/3
  Compare To Constant1/1 -> Logical Operator/1
  Compare To Constant8/1 -> Logical Operator/2
  Logical Operator/1 -> Logical Operator1/2
  FCW_AEB_JAFctSts/1 -> Compare To Constant9/1, Compare To Constant8/1, Compare To Constant1/1

== system TosAeb/FcwAebJa_Step_sys/ALG_AEBSystem/ALG_AEBController/AEB_VehicleStateCalcApp/JaSuppAborCondtionCalc/JAPedAEBSuppAborConditonCalc/VehStateCalcJAAEBSuppCalc ==
  AEB_PedResp : Inport
  FCW_AEB_JAFctSts : Inport  Port=2
  vehUnstableStateFlg : Inport  Port=3
  vehAssociatedSysStateSuppCond : Inport  Port=4
  "VehicleConfigAEBPed " : Inport  Port=5
  blockTimeBrake : Inport  Port=6
  Compare To Constant : simulink/Logic and Bit Operations/Compare To Constant  relop===  const=CNSwtOFF  OutDataTypeStr=boolean
  Compare To Constant1 : simulink/Logic and Bit Operations/Compare To Constant  relop===  const=CN_VSC_FCW_AEB_Error  OutDataTypeStr=boolean
  Compare To Constant2 : simulink/Logic and Bit Operations/Compare To Constant  relop===  const=TRUE  OutDataTypeStr=boolean
  Compare To Constant3 : simulink/Logic and Bit Operations/Compare To Constant  relop===  const=CNVehConfigAEBPedNone  OutDataTypeStr=boolean
  Compare To Constant4 : simulink/Logic and Bit Operations/Compare To Constant  relop=>  const=CNNull  OutDataTypeStr=boolean
  Compare To Constant5 : simulink/Logic and Bit Operations/Compare To Constant  relop===  const=TRUE  OutDataTypeStr=boolean
  Compare To Constant7 : simulink/Logic and Bit Operations/Compare To Constant  relop===  const=CNSwtOFF  OutDataTypeStr=boolean
  Compare To Constant8 : simulink/Logic and Bit Operations/Compare To Constant  relop===  const=CN_VSC_FCW_AEB_PerfmDgdtn  OutDataTypeStr=boolean
  Compare To Constant9 : simulink/Logic and Bit Operations/Compare To Constant  relop===  const=CN_VSC_FCW_AEB_TempUnAvlbl  OutDataTypeStr=boolean
  Constant : Constant  Value=P_AEB_InterSwtSts4JAPedAEBfull
  Logical Operator : Logic  Operator=OR  Inputs=3  AllPortsSameDT=off  OutDataTypeStr=boolean
  Logical Operator1 : Logic  Operator=OR  Inputs=7  AllPortsSameDT=off  OutDataTypeStr=boolean
  VSCJAPedAEBfullSuppCond : Outport
  wires:
  Compare To Constant4/1 -> Logical Operator1/6
  "VehicleConfigAEBPed "/1 -> Compare To Constant3/1
  Logical Operator1/1 -> VSCJAPedAEBfullSuppCond/1
  vehUnstableStateFlg/1 -> Compare To Constant2/1
  blockTimeBrake/1 -> Compare To Constant4/1
  vehAssociatedSysStateSuppCond/1 -> Compare To Constant5/1
  Compare To Constant3/1 -> Logical Operator1/5
  Compare To Constant/1 -> Logical Operator1/1
  AEB_PedResp/1 -> Compare To Constant/1
  Compare To Constant2/1 -> Logical Operator1/3
  Compare To Constant5/1 -> Logical Operator1/4
  Constant/1 -> Compare To Constant7/1
  Compare To Constant7/1 -> Logical Operator1/7
  Compare To Constant9/1 -> Logical Operator/3
  Compare To Constant1/1 -> Logical Operator/1
  Compare To Constant8/1 -> Logical Operator/2
  Logical Operator/1 -> Logical Operator1/2
  FCW_AEB_JAFctSts/1 -> Compare To Constant9/1, Compare To Constant8/1, Compare To Constant1/1

== system TosAeb/FcwAebJa_Step_sys/ALG_AEBSystem/ALG_AEBController/AEB_VehicleStateCalcApp/JaSuppAborCondtionCalc/JAPedPrefillSuppAborConditonCalc/VehStateCalcJAPrefillAborCalc ==
  AEB_PedResp : Inport
  FCW_AEB_JAFctSts : Inport  Port=2
  vehAssociatedSysStateSuppCond : Inport  Port=3
  vehUnstableStateFlg : Inport  Port=4
  Compare To Constant : simulink/Logic and Bit Operations/Compare To Constant  relop===  const=CNSwtOFF  OutDataTypeStr=boolean
  Compare To Constant1 : simulink/Logic and Bit Operations/Compare To Constant  relop===  const=CN_VSC_FCW_AEB_Error  OutDataTypeStr=boolean
  Compare To Constant2 : simulink/Logic and Bit Operations/Compare To Constant  relop===  const=TRUE  OutDataTypeStr=boolean
  Compare To Constant3 : simulink/Logic and Bit Operations/Compare To Constant  relop===  const=TRUE  OutDataTypeStr=boolean
  Compare To Constant8 : simulink/Logic and Bit Operations/Compare To Constant  relop===  const=CN_VSC_FCW_AEB_PerfmDgdtn  OutDataTypeStr=boolean
  Compare To Constant9 : simulink/Logic and Bit Operations/Compare To Constant  relop===  const=CN_VSC_FCW_AEB_TempUnAvlbl  OutDataTypeStr=boolean
  Logical Operator : Logic  Operator=OR  Inputs=3  AllPortsSameDT=off  OutDataTypeStr=boolean
  Logical Operator1 : Logic  Operator=OR  Inputs=4  AllPortsSameDT=off  OutDataTypeStr=boolean
  VSCJAPedPrefillAborCond : Outport
  wires:
  vehUnstableStateFlg/1 -> Compare To Constant3/1
  Logical Operator1/1 -> VSCJAPedPrefillAborCond/1
  vehAssociatedSysStateSuppCond/1 -> Compare To Constant2/1
  Compare To Constant/1 -> Logical Operator1/1
  AEB_PedResp/1 -> Compare To Constant/1
  Compare To Constant2/1 -> Logical Operator1/3
  Compare To Constant3/1 -> Logical Operator1/4
  Compare To Constant9/1 -> Logical Operator/3
  Compare To Constant1/1 -> Logical Operator/1
  Compare To Constant8/1 -> Logical Operator/2
  Logical Operator/1 -> Logical Operator1/2
  FCW_AEB_JAFctSts/1 -> Compare To Constant9/1, Compare To Constant8/1, Compare To Constant1/1

== system TosAeb/FcwAebJa_Step_sys/ALG_AEBSystem/ALG_AEBController/AEB_VehicleStateCalcApp/JaSuppAborCondtionCalc/JAPedPrefillSuppAborConditonCalc/VehStateCalcJAPrefillSuppCalc ==
  AEB_PedResp : Inport
  FCW_AEB_JAFctSts : Inport  Port=2
  vehUnstableStateFlg : Inport  Port=3
  vehAssociatedSysStateSuppCond : Inport  Port=4
  "VehicleConfigAEBPed " : Inport  Port=5
  blockTimeBrakeReady : Inport  Port=6
  Compare To Constant : simulink/Logic and Bit Operations/Compare To Constant  relop===  const=CNSwtOFF  OutDataTypeStr=boolean
  Compare To Constant1 : simulink/Logic and Bit Operations/Compare To Constant  relop===  const=CN_VSC_FCW_AEB_Error  OutDataTypeStr=boolean
  Compare To Constant3 : simulink/Logic and Bit Operations/Compare To Constant  relop===  const=CNVehConfigAEBPedNone  OutDataTypeStr=boolean
  Compare To Constant4 : simulink/Logic and Bit Operations/Compare To Constant  relop=>  const=CNNull  OutDataTypeStr=boolean
  Compare To Constant5 : simulink/Logic and Bit Operations/Compare To Constant  relop===  const=TRUE  OutDataTypeStr=boolean
  Compare To Constant6 : simulink/Logic and Bit Operations/Compare To Constant  relop===  const=TRUE  OutDataTypeStr=boolean
  Compare To Constant7 : simulink/Logic and Bit Operations/Compare To Constant  relop===  const=CNSwtOFF  OutDataTypeStr=boolean
  Compare To Constant8 : simulink/Logic and Bit Operations/Compare To Constant  relop===  const=CN_VSC_FCW_AEB_PerfmDgdtn  OutDataTypeStr=boolean
  Compare To Constant9 : simulink/Logic and Bit Operations/Compare To Constant  relop===  const=CN_VSC_FCW_AEB_TempUnAvlbl  OutDataTypeStr=boolean
  Constant : Constant  Value=P_AEB_InterSwtSts4JAPedPrefill
  Logical Operator : Logic  Operator=OR  Inputs=3  AllPortsSameDT=off  OutDataTypeStr=boolean
  Logical Operator1 : Logic  Operator=OR  Inputs=6  AllPortsSameDT=off  OutDataTypeStr=boolean
  Terminator : Terminator
  VSCJAPedPrefillSuppCond : Outport
  wires:
  AEB_PedResp/1 -> Compare To Constant/1
  Compare To Constant/1 -> Logical Operator1/1
  Compare To Constant3/1 -> Logical Operator1/5
  vehAssociatedSysStateSuppCond/1 -> Compare To Constant5/1
  blockTimeBrakeReady/1 -> Compare To Constant4/1
  vehUnstableStateFlg/1 -> Compare To Constant6/1
  Logical Operator1/1 -> VSCJAPedPrefillSuppCond/1
  "VehicleConfigAEBPed "/1 -> Compare To Constant3/1
  Compare To Constant4/1 -> Terminator/1
  Compare To Constant5/1 -> Logical Operator1/4
  Compare To Constant6/1 -> Logical Operator1/3
  Constant/1 -> Compare To Constant7/1
  Compare To Constant7/1 -> Logical Operator1/6
  Compare To Constant9/1 -> Logical Operator/3
  Compare To Constant1/1 -> Logical Operator/1
  Compare To Constant8/1 -> Logical Operator/2
  Logical Operator/1 -> Logical Operator1/2
  FCW_AEB_JAFctSts/1 -> Compare To Constant9/1, Compare To Constant8/1, Compare To Constant1/1

== system TosAeb/FcwAebJa_Step_sys/ALG_AEBSystem/ALG_AEBController/AEB_VehicleStateCalcApp/JaSuppAborCondtionCalc/JAVehAEBSuppAborConditonCalc/VehStateCalcJAAEBAborCalc ==
  VSCAEB_JAResp : Inport
  FCW_AEB_JAFctSts : Inport  Port=2
  vehAssociatedSysStateSuppCond : Inport  Port=3
  vehUnstableStateFlg : Inport  Port=4
  Compare To Constant : simulink/Logic and Bit Operations/Compare To Constant  relop===  const=CNSwtOFF  OutDataTypeStr=boolean
  Compare To Constant1 : simulink/Logic and Bit Operations/Compare To Constant  relop===  const=CN_VSC_FCW_AEB_Error  OutDataTypeStr=boolean
  Compare To Constant2 : simulink/Logic and Bit Operations/Compare To Constant  relop===  const=TRUE  OutDataTypeStr=boolean
  Compare To Constant3 : simulink/Logic and Bit Operations/Compare To Constant  relop===  const=TRUE  OutDataTypeStr=boolean
  Compare To Constant8 : simulink/Logic and Bit Operations/Compare To Constant  relop===  const=CN_VSC_FCW_AEB_PerfmDgdtn  OutDataTypeStr=boolean
  Compare To Constant9 : simulink/Logic and Bit Operations/Compare To Constant  relop===  const=CN_VSC_FCW_AEB_TempUnAvlbl  OutDataTypeStr=boolean
  Logical Operator : Logic  Operator=OR  Inputs=3  AllPortsSameDT=off  OutDataTypeStr=boolean
  Logical Operator1 : Logic  Operator=OR  Inputs=4  AllPortsSameDT=off  OutDataTypeStr=boolean
  VSCJAVehAEBAborCond : Outport
  wires:
  Compare To Constant3/1 -> Logical Operator1/4
  Compare To Constant2/1 -> Logical Operator1/3
  vehUnstableStateFlg/1 -> Compare To Constant3/1
  Logical Operator1/1 -> VSCJAVehAEBAborCond/1
  vehAssociatedSysStateSuppCond/1 -> Compare To Constant2/1
  Compare To Constant/1 -> Logical Operator1/1
  VSCAEB_JAResp/1 -> Compare To Constant/1
  Compare To Constant1/1 -> Logical Operator/1
  Compare To Constant8/1 -> Logical Operator/2
  Compare To Constant9/1 -> Logical Operator/3
  Logical Operator/1 -> Logical Operator1/2
  FCW_AEB_JAFctSts/1 -> Compare To Constant9/1, Compare To Constant8/1, Compare To Constant1/1

== system TosAeb/FcwAebJa_Step_sys/ALG_AEBSystem/ALG_AEBController/AEB_VehicleStateCalcApp/JaSuppAborCondtionCalc/JAVehAEBSuppAborConditonCalc/VehStateCalcJAAEBSuppCalc ==
  VSCAEB_JAResp : Inport
  FCW_AEB_JAFctSts : Inport  Port=2
  vehUnstableStateFlg : Inport  Port=3
  vehAssociatedSysStateSuppCond : Inport  Port=4
  "vehConfigAEBJA " : Inport  Port=5
  blockTimeBrake : Inport  Port=6
  Compare To Constant : simulink/Logic and Bit Operations/Compare To Constant  relop===  const=CNSwtOFF  OutDataTypeStr=boolean
  Compare To Constant1 : simulink/Logic and Bit Operations/Compare To Constant  relop===  const=CN_VSC_FCW_AEB_Error  OutDataTypeStr=boolean
  Compare To Constant2 : simulink/Logic and Bit Operations/Compare To Constant  relop===  const=TRUE  OutDataTypeStr=boolean
  Compare To Constant3 : simulink/Logic and Bit Operations/Compare To Constant  relop===  const=CNVehConfigJANone  OutDataTypeStr=boolean
  Compare To Constant4 : simulink/Logic and Bit Operations/Compare To Constant  relop=>  const=CNNull  OutDataTypeStr=boolean
  Compare To Constant5 : simulink/Logic and Bit Operations/Compare To Constant  relop===  const=TRUE  OutDataTypeStr=boolean
  Compare To Constant7 : simulink/Logic and Bit Operations/Compare To Constant  relop===  const=CNSwtOFF  OutDataTypeStr=boolean
  Compare To Constant8 : simulink/Logic and Bit Operations/Compare To Constant  relop===  const=CN_VSC_FCW_AEB_PerfmDgdtn  OutDataTypeStr=boolean
  Compare To Constant9 : simulink/Logic and Bit Operations/Compare To Constant  relop===  const=CN_VSC_FCW_AEB_TempUnAvlbl  OutDataTypeStr=boolean
  Constant : Constant  Value=P_AEB_InterSwtSts4JAVehAEBfull
  Logical Operator : Logic  Operator=OR  Inputs=3  AllPortsSameDT=off  OutDataTypeStr=boolean
  Logical Operator1 : Logic  Operator=OR  Inputs=7  AllPortsSameDT=off  OutDataTypeStr=boolean
  VSCJAVehAEBfullSuppCond : Outport
  wires:
  Compare To Constant7/1 -> Logical Operator1/7
  Constant/1 -> Compare To Constant7/1
  Compare To Constant5/1 -> Logical Operator1/4
  Compare To Constant2/1 -> Logical Operator1/3
  VSCAEB_JAResp/1 -> Compare To Constant/1
  Compare To Constant/1 -> Logical Operator1/1
  Compare To Constant3/1 -> Logical Operator1/5
  vehAssociatedSysStateSuppCond/1 -> Compare To Constant5/1
  blockTimeBrake/1 -> Compare To Constant4/1
  vehUnstableStateFlg/1 -> Compare To Constant2/1
  Logical Operator1/1 -> VSCJAVehAEBfullSuppCond/1
  "vehConfigAEBJA "/1 -> Compare To Constant3/1
  Compare To Constant4/1 -> Logical Operator1/6
  Compare To Constant1/1 -> Logical Operator/1
  Compare To Constant8/1 -> Logical Operator/2
  Compare To Constant9/1 -> Logical Operator/3
  Logical Operator/1 -> Logical Operator1/2
  FCW_AEB_JAFctSts/1 -> Compare To Constant9/1, Compare To Constant8/1, Compare To Constant1/1
